simulink model "siento3"  (Simulink 7.2)
settings: Solver=ode45  StartTime=0.0  StopTime=10.0  RelTol=1e-3
undefined symbols (not defined in the file or in its folder): ecgGen, emgGen, fdesignblkfcn, gcbh, scGen
libraries: dspsigops x684, dspbuff3 x3, dspsrcs4 x3
(part 5 of 5)

== matlab function Embedded MATLAB Function1 ==
  data: data input double; featvec output double; featnames output double
function [featvec,featnames] = aubt_proxy_emg(data)
% This block supports the Embedded MATLAB subset.
% See the help menu for details. 
hz=1000;
%[featvec, featnames]=aubt_extractFeatSC(data,hz);

%testing setup
featvec=[sum(data);rand(3,1)];
%simulink doesn't support string or char outputs, so convert to int
featnames=uint8(['feat1'; 'feat2'; 'feat3'; 'feat4']);

== matlab function Embedded MATLAB Function ==
  data: data input double; featvec output double; featnames output double
function [featvec,featnames]  = aubt_proxy_ecg_2(data)
% This block supports the Embedded MATLAB subset.
% See the help menu for details. 
hz=1000;
%[featvec, featnames]=aubt_extractFeatECG(data,hz);

%testing setup
featvec=[sum(data);rand(3,1)];
%simulink doesn't support string or char outputs, so convert to int
featnames=uint8(['feat1'; 'feat2'; 'feat3'; 'feat4']);

== matlab function Embedded MATLAB Function2 ==
  data: data input double; featvec output double; featnames output double
function [featvec, featnames] = aubt_proxy_ecg_2(data)
% This block supports the Embedded MATLAB subset.
% See the help menu for details. 
hz=1000;
%[featvec, featnames]=aubt_extractFeatECG(data,hz);

%testing setup
featvec=[sum(data);rand(3,1)];
%simulink doesn't support string or char outputs, so convert to int
featnames=uint8(['feat1'; 'feat2'; 'feat3'; 'feat4']);

== matlab function Embedded MATLAB Function3 ==
  data: featmat input double; labels input double
function  fisherPlot(featmat, labels)
% This block supports the Embedded MATLAB subset.
% See the help menu for details. 

eml.extrinsic('aubt_fisherPlot');
aubt_fisherPlot(featmat,labels,'r',3,[]);
